simulink model "cddDcmApp"  (R2022b)
settings: Solver=FixedStepDiscrete  StartTime=0.0  StopTime=10.0  FixedStep=0.02  RelTol=1e-3
undefined symbols (not defined in the file or in its folder): CCPFaultType, DcChlType, HFAChlType, IcuChannelType, MtrRoutStaEnum, P_SignalPartType, P_Std_ReturnType, PowerChlType, SrsAdChlType, VGAChlType
(part 5 of 13)

== system cddDcmApp/cddDcmApp_Init_1 ==
  Event Listener : EventListener  EventName=reset

== system cddDcmApp/cddDcmApp_Init_sys ==
  function : TriggerPort  TriggerType=function-call  VariantControl=(inherit)

== system cddDcmApp/DataServices_DID_4756_DID_4756_Front_Mosfet_Blower_FB/DataServices_DID_4756_DID_4756_Front_Mosfet_Blower_FB_Voltage_sys ==
  function : TriggerPort  TriggerType=function-call  IsSimulinkFunction=on  FunctionName=DataServices_DID_4756_DID_4756_Front_Mosfet_Blower_FB_Voltage  VariantControl=(inherit)
  Ground_1 : Ground
  Ground_2 : Ground
  Terminator_1 : Terminator
  OpStatus : ArgIn  OutDataTypeStr=Enum: Dcm_OpStatusType  ArgumentName=OpStatus
  Data : ArgOut  OutDataTypeStr=uint8  ArgumentName=Data
  ERR : ArgOut  Port=2  OutDataTypeStr=Std_ReturnType  ArgumentName=ERR
  wires:
  OpStatus/1 -> Terminator_1/1
  Ground_1/1 -> Data/1
  Ground_2/1 -> ERR/1

== system cddDcmApp/DataServices_DID_47F3_DID_47F3_AIU_Failure_Reasons_and/DataServices_DID_47F3_DID_47F3_AIU_Failure_Reasons_and_Status_sys ==
  function : TriggerPort  TriggerType=function-call  IsSimulinkFunction=on  FunctionName=DataServices_DID_47F3_DID_47F3_AIU_Failure_Reasons_and_Status  VariantControl=(inherit)
  Ground_1 : Ground
  Ground_2 : Ground
  Terminator_1 : Terminator
  OpStatus : ArgIn  OutDataTypeStr=Enum: Dcm_OpStatusType  ArgumentName=OpStatus
  Data : ArgOut  OutDataTypeStr=uint8  ArgumentName=Data
  ERR : ArgOut  Port=2  OutDataTypeStr=Std_ReturnType  ArgumentName=ERR
  wires:
  OpStatus/1 -> Terminator_1/1
  Ground_1/1 -> Data/1
  Ground_2/1 -> ERR/1

== system cddDcmApp/DataServices_DID_47F4_DID_47F4_AQS_Air_Quality/DataServices_DID_47F4_DID_47F4_AQS_Air_Quality_Level_sys ==
  function : TriggerPort  TriggerType=function-call  IsSimulinkFunction=on  FunctionName=DataServices_DID_47F4_DID_47F4_AQS_Air_Quality_Level  VariantControl=(inherit)
  Ground_1 : Ground
  Ground_2 : Ground
  Terminator_1 : Terminator
  OpStatus : ArgIn  OutDataTypeStr=Enum: Dcm_OpStatusType  ArgumentName=OpStatus
  Data : ArgOut  OutDataTypeStr=uint8  ArgumentName=Data
  ERR : ArgOut  Port=2  OutDataTypeStr=Std_ReturnType  ArgumentName=ERR
  wires:
  OpStatus/1 -> Terminator_1/1
  Ground_1/1 -> Data/1
  Ground_2/1 -> ERR/1

== system cddDcmApp/DataServices_DID_47F5_DID_47F5_TestRunCriteria_TRC/DataServices_DID_47F5_DID_47F5_TestRunCriteria_TRC_Result_sys ==
  function : TriggerPort  TriggerType=function-call  IsSimulinkFunction=on  FunctionName=DataServices_DID_47F5_DID_47F5_TestRunCriteria_TRC_Result  VariantControl=(inherit)
  Ground_1 : Ground
  Ground_2 : Ground
  Terminator_1 : Terminator
  OpStatus : ArgIn  OutDataTypeStr=Enum: Dcm_OpStatusType  ArgumentName=OpStatus
  Data : ArgOut  OutDataTypeStr=uint8  ArgumentName=Data
  ERR : ArgOut  Port=2  OutDataTypeStr=Std_ReturnType  ArgumentName=ERR
  wires:
  OpStatus/1 -> Terminator_1/1
  Ground_1/1 -> Data/1
  Ground_2/1 -> ERR/1

== system cddDcmApp/DataServices_DID_47F6_DID_47F6_CCM_Lin1_Frame/DataServices_DID_47F6_DID_47F6_CCM_Lin1_Frame_Status_sys ==
  function : TriggerPort  TriggerType=function-call  IsSimulinkFunction=on  FunctionName=DataServices_DID_47F6_DID_47F6_CCM_Lin1_Frame_Status  VariantControl=(inherit)
  Ground_1 : Ground
  Ground_2 : Ground
  Terminator_1 : Terminator
  OpStatus : ArgIn  OutDataTypeStr=Enum: Dcm_OpStatusType  ArgumentName=OpStatus
  Data : ArgOut  OutDataTypeStr=uint8  ArgumentName=Data
  ERR : ArgOut  Port=2  OutDataTypeStr=Std_ReturnType  ArgumentName=ERR
  wires:
  OpStatus/1 -> Terminator_1/1
  Ground_1/1 -> Data/1
  Ground_2/1 -> ERR/1

== system cddDcmApp/DataServices_DID_47F7_DID_47F7_CCM_Lin2_Frame/DataServices_DID_47F7_DID_47F7_CCM_Lin2_Frame_Status_sys ==
  function : TriggerPort  TriggerType=function-call  IsSimulinkFunction=on  FunctionName=DataServices_DID_47F7_DID_47F7_CCM_Lin2_Frame_Status  VariantControl=(inherit)
  Ground_1 : Ground
  Ground_2 : Ground
  Terminator_1 : Terminator
  OpStatus : ArgIn  OutDataTypeStr=Enum: Dcm_OpStatusType  ArgumentName=OpStatus
  Data : ArgOut  OutDataTypeStr=uint8  ArgumentName=Data
  ERR : ArgOut  Port=2  OutDataTypeStr=Std_ReturnType  ArgumentName=ERR
  wires:
  OpStatus/1 -> Terminator_1/1
  Ground_1/1 -> Data/1
  Ground_2/1 -> ERR/1

== system cddDcmApp/DataServices_DID_47F8_DID_47F8_CCM_Lin3_Frame/DataServices_DID_47F8_DID_47F8_CCM_Lin3_Frame_Status_sys ==
  function : TriggerPort  TriggerType=function-call  IsSimulinkFunction=on  FunctionName=DataServices_DID_47F8_DID_47F8_CCM_Lin3_Frame_Status  VariantControl=(inherit)
  Ground_1 : Ground
  Ground_2 : Ground
  Terminator_1 : Terminator
  OpStatus : ArgIn  OutDataTypeStr=Enum: Dcm_OpStatusType  ArgumentName=OpStatus
  Data : ArgOut  OutDataTypeStr=uint8  ArgumentName=Data
  ERR : ArgOut  Port=2  OutDataTypeStr=Std_ReturnType  ArgumentName=ERR
  wires:
  OpStatus/1 -> Terminator_1/1
  Ground_1/1 -> Data/1
  Ground_2/1 -> ERR/1

== system cddDcmApp/DataServices_DID_47F9_DID_47F9_Blower_Max_Level_Voltage/DataServices_DID_47F9_DID_47F9_Blower_Max_Level_Voltage_Limit_sys ==
  function : TriggerPort  TriggerType=function-call  IsSimulinkFunction=on  FunctionName=DataServices_DID_47F9_DID_47F9_Blower_Max_Level_Voltage_Limit  VariantControl=(inherit)
  Ground_1 : Ground
  Ground_2 : Ground
  Terminator_1 : Terminator
  OpStatus : ArgIn  OutDataTypeStr=Enum: Dcm_OpStatusType  ArgumentName=OpStatus
  Data : ArgOut  OutDataTypeStr=uint8  ArgumentName=Data
  ERR : ArgOut  Port=2  OutDataTypeStr=Std_ReturnType  ArgumentName=ERR
  wires:
  OpStatus/1 -> Terminator_1/1
  Ground_1/1 -> Data/1
  Ground_2/1 -> ERR/1

== system cddDcmApp/DataServices_DID_4AF7_DID_4AF7_1_DC_Motor_Drive_Request_Number/DataServices_DID_4AF7_DID_4AF7_1_DC_Motor_Drive_Request_Number_FreezeCurrentState_sys ==
  function : TriggerPort  TriggerType=function-call  IsSimulinkFunction=on  FunctionName=DataServices_DID_4AF7_DID_4AF7_1_DC_Motor_Drive_Request_Number_FreezeCurrentState  VariantControl=(inherit)
  Ground_1 : Ground
  Ground_2 : Ground
  Terminator_1 : Terminator
  OpStatus : ArgIn  OutDataTypeStr=Enum: Dcm_OpStatusType  ArgumentName=OpStatus
  ErrorCode : ArgOut  OutDataTypeStr=Enum: Dcm_NegativeResponseCodeType  ArgumentName=ErrorCode
  ERR : ArgOut  Port=2  OutDataTypeStr=Std_ReturnType  ArgumentName=ERR
  wires:
  OpStatus/1 -> Terminator_1/1
  Ground_1/1 -> ErrorCode/1
  Ground_2/1 -> ERR/1

== system cddDcmApp/DataServices_DID_4AF7_DID_4AF7_1_DC_Motor_Drive_Request_Number/DataServices_DID_4AF7_DID_4AF7_1_DC_Motor_Drive_Request_Number_ReadData_sys ==
  function : TriggerPort  TriggerType=function-call  IsSimulinkFunction=on  FunctionName=DataServices_DID_4AF7_DID_4AF7_1_DC_Motor_Drive_Request_Number_ReadData  VariantControl=(inherit)
  Ground_1 : Ground
  Ground_2 : Ground
  Terminator_1 : Terminator
  OpStatus : ArgIn  OutDataTypeStr=Enum: Dcm_OpStatusType  ArgumentName=OpStatus
  Data : ArgOut  OutDataTypeStr=uint8  ArgumentName=Data
  ERR : ArgOut  Port=2  OutDataTypeStr=Std_ReturnType  ArgumentName=ERR
  wires:
  OpStatus/1 -> Terminator_1/1
  Ground_1/1 -> Data/1
  Ground_2/1 -> ERR/1

== system cddDcmApp/DataServices_DID_4AF7_DID_4AF7_1_DC_Motor_Drive_Request_Number/DataServices_DID_4AF7_DID_4AF7_1_DC_Motor_Drive_Request_Number_ReturnControlToECU_sys ==
  function : TriggerPort  TriggerType=function-call  IsSimulinkFunction=on  FunctionName=DataServices_DID_4AF7_DID_4AF7_1_DC_Motor_Drive_Request_Number_ReturnControlToECU  VariantControl=(inherit)
  Ground_1 : Ground
  Ground_2 : Ground
  ErrorCode : ArgOut  OutDataTypeStr=Enum: Dcm_NegativeResponseCodeType  ArgumentName=ErrorCode
  ERR : ArgOut  Port=2  OutDataTypeStr=Std_ReturnType  ArgumentName=ERR
  wires:
  Ground_1/1 -> ErrorCode/1
  Ground_2/1 -> ERR/1

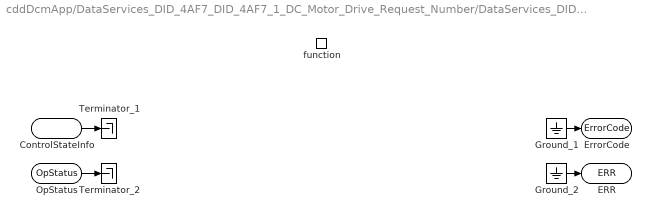
== system cddDcmApp/DataServices_DID_4AF7_DID_4AF7_1_DC_Motor_Drive_Request_Number/DataServices_DID_4AF7_DID_4AF7_1_DC_Motor_Drive_Request_Number_ShortTermAdjustment_sys ==
  function : TriggerPort  TriggerType=function-call  IsSimulinkFunction=on  FunctionName=DataServices_DID_4AF7_DID_4AF7_1_DC_Motor_Drive_Request_Number_ShortTermAdjustment  VariantControl=(inherit)
  Ground_1 : Ground
  Ground_2 : Ground
  Terminator_1 : Terminator
  Terminator_2 : Terminator
  ControlStateInfo : ArgIn  OutDataTypeStr=uint8  ArgumentName=ControlStateInfo
  OpStatus : ArgIn  Port=2  OutDataTypeStr=Enum: Dcm_OpStatusType  ArgumentName=OpStatus
  ErrorCode : ArgOut  OutDataTypeStr=Enum: Dcm_NegativeResponseCodeType  ArgumentName=ErrorCode
  ERR : ArgOut  Port=2  OutDataTypeStr=Std_ReturnType  ArgumentName=ERR
  wires:
  ControlStateInfo/1 -> Terminator_1/1
  OpStatus/1 -> Terminator_2/1
  Ground_1/1 -> ErrorCode/1
  Ground_2/1 -> ERR/1

== system cddDcmApp/DataServices_DID_4AF7_DID_4AF7_2_DC_Motor_Drive_Request_Time/DataServices_DID_4AF7_DID_4AF7_2_DC_Motor_Drive_Request_Time_FreezeCurrentState_sys ==
  function : TriggerPort  TriggerType=function-call  IsSimulinkFunction=on  FunctionName=DataServices_DID_4AF7_DID_4AF7_2_DC_Motor_Drive_Request_Time_FreezeCurrentState  VariantControl=(inherit)
  Ground_1 : Ground
  Ground_2 : Ground
  Terminator_1 : Terminator
  OpStatus : ArgIn  OutDataTypeStr=Enum: Dcm_OpStatusType  ArgumentName=OpStatus
  ErrorCode : ArgOut  OutDataTypeStr=Enum: Dcm_NegativeResponseCodeType  ArgumentName=ErrorCode
  ERR : ArgOut  Port=2  OutDataTypeStr=Std_ReturnType  ArgumentName=ERR
  wires:
  OpStatus/1 -> Terminator_1/1
  Ground_1/1 -> ErrorCode/1
  Ground_2/1 -> ERR/1

== system cddDcmApp/DataServices_DID_4AF7_DID_4AF7_2_DC_Motor_Drive_Request_Time/DataServices_DID_4AF7_DID_4AF7_2_DC_Motor_Drive_Request_Time_ReadData_sys ==
  function : TriggerPort  TriggerType=function-call  IsSimulinkFunction=on  FunctionName=DataServices_DID_4AF7_DID_4AF7_2_DC_Motor_Drive_Request_Time_ReadData  VariantControl=(inherit)
  Ground_1 : Ground
  Ground_2 : Ground
  Terminator_1 : Terminator
  OpStatus : ArgIn  OutDataTypeStr=Enum: Dcm_OpStatusType  ArgumentName=OpStatus
  Data : ArgOut  OutDataTypeStr=uint8  ArgumentName=Data
  ERR : ArgOut  Port=2  OutDataTypeStr=Std_ReturnType  ArgumentName=ERR
  wires:
  OpStatus/1 -> Terminator_1/1
  Ground_1/1 -> Data/1
  Ground_2/1 -> ERR/1

== system cddDcmApp/DataServices_DID_4AF7_DID_4AF7_2_DC_Motor_Drive_Request_Time/DataServices_DID_4AF7_DID_4AF7_2_DC_Motor_Drive_Request_Time_ReturnControlToECU_sys ==
  function : TriggerPort  TriggerType=function-call  IsSimulinkFunction=on  FunctionName=DataServices_DID_4AF7_DID_4AF7_2_DC_Motor_Drive_Request_Time_ReturnControlToECU  VariantControl=(inherit)
  Ground_1 : Ground
  Ground_2 : Ground
  ErrorCode : ArgOut  OutDataTypeStr=Enum: Dcm_NegativeResponseCodeType  ArgumentName=ErrorCode
  ERR : ArgOut  Port=2  OutDataTypeStr=Std_ReturnType  ArgumentName=ERR
  wires:
  Ground_1/1 -> ErrorCode/1
  Ground_2/1 -> ERR/1

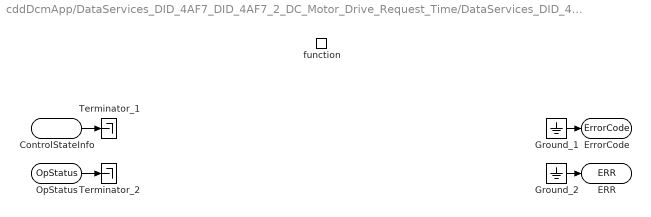
== system cddDcmApp/DataServices_DID_4AF7_DID_4AF7_2_DC_Motor_Drive_Request_Time/DataServices_DID_4AF7_DID_4AF7_2_DC_Motor_Drive_Request_Time_ShortTermAdjustment_sys ==
  function : TriggerPort  TriggerType=function-call  IsSimulinkFunction=on  FunctionName=DataServices_DID_4AF7_DID_4AF7_2_DC_Motor_Drive_Request_Time_ShortTermAdjustment  VariantControl=(inherit)
  Ground_1 : Ground
  Ground_2 : Ground
  Terminator_1 : Terminator
  Terminator_2 : Terminator
  ControlStateInfo : ArgIn  OutDataTypeStr=uint8  ArgumentName=ControlStateInfo
  OpStatus : ArgIn  Port=2  OutDataTypeStr=Enum: Dcm_OpStatusType  ArgumentName=OpStatus
  ErrorCode : ArgOut  OutDataTypeStr=Enum: Dcm_NegativeResponseCodeType  ArgumentName=ErrorCode
  ERR : ArgOut  Port=2  OutDataTypeStr=Std_ReturnType  ArgumentName=ERR
  wires:
  ControlStateInfo/1 -> Terminator_1/1
  OpStatus/1 -> Terminator_2/1
  Ground_1/1 -> ErrorCode/1
  Ground_2/1 -> ERR/1

== system cddDcmApp/DataServices_DID_4AF7_DID_4AF7_3_DC_Motor_Drive_Request_Direction/DataServices_DID_4AF7_DID_4AF7_3_DC_Motor_Drive_Request_Direction_FreezeCurrentState_sys ==
  function : TriggerPort  TriggerType=function-call  IsSimulinkFunction=on  FunctionName=DataServices_DID_4AF7_DID_4AF7_3_DC_Motor_Drive_Request_Direction_FreezeCurrentState  VariantControl=(inherit)
  Ground_1 : Ground
  Ground_2 : Ground
  Terminator_1 : Terminator
  OpStatus : ArgIn  OutDataTypeStr=Enum: Dcm_OpStatusType  ArgumentName=OpStatus
  ErrorCode : ArgOut  OutDataTypeStr=Enum: Dcm_NegativeResponseCodeType  ArgumentName=ErrorCode
  ERR : ArgOut  Port=2  OutDataTypeStr=Std_ReturnType  ArgumentName=ERR
  wires:
  OpStatus/1 -> Terminator_1/1
  Ground_1/1 -> ErrorCode/1
  Ground_2/1 -> ERR/1

== system cddDcmApp/DataServices_DID_4AF7_DID_4AF7_3_DC_Motor_Drive_Request_Direction/DataServices_DID_4AF7_DID_4AF7_3_DC_Motor_Drive_Request_Direction_ReadData_sys ==
  function : TriggerPort  TriggerType=function-call  IsSimulinkFunction=on  FunctionName=DataServices_DID_4AF7_DID_4AF7_3_DC_Motor_Drive_Request_Direction_ReadData  VariantControl=(inherit)
  Ground_1 : Ground
  Ground_2 : Ground
  Terminator_1 : Terminator
  OpStatus : ArgIn  OutDataTypeStr=Enum: Dcm_OpStatusType  ArgumentName=OpStatus
  Data : ArgOut  OutDataTypeStr=uint8  ArgumentName=Data
  ERR : ArgOut  Port=2  OutDataTypeStr=Std_ReturnType  ArgumentName=ERR
  wires:
  OpStatus/1 -> Terminator_1/1
  Ground_1/1 -> Data/1
  Ground_2/1 -> ERR/1

== system cddDcmApp/DataServices_DID_4AF7_DID_4AF7_3_DC_Motor_Drive_Request_Direction/DataServices_DID_4AF7_DID_4AF7_3_DC_Motor_Drive_Request_Direction_ReturnControlToECU_sys ==
  function : TriggerPort  TriggerType=function-call  IsSimulinkFunction=on  FunctionName=DataServices_DID_4AF7_DID_4AF7_3_DC_Motor_Drive_Request_Direction_ReturnControlToECU  VariantControl=(inherit)
  Ground_1 : Ground
  Ground_2 : Ground
  ErrorCode : ArgOut  OutDataTypeStr=Enum: Dcm_NegativeResponseCodeType  ArgumentName=ErrorCode
  ERR : ArgOut  Port=2  OutDataTypeStr=Std_ReturnType  ArgumentName=ERR
  wires:
  Ground_1/1 -> ErrorCode/1
  Ground_2/1 -> ERR/1

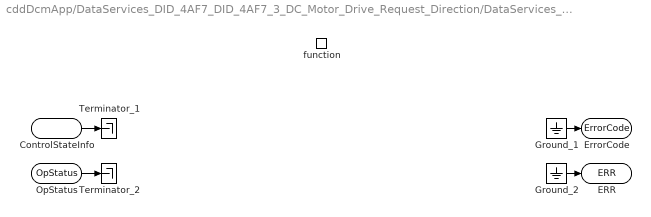
== system cddDcmApp/DataServices_DID_4AF7_DID_4AF7_3_DC_Motor_Drive_Request_Direction/DataServices_DID_4AF7_DID_4AF7_3_DC_Motor_Drive_Request_Direction_ShortTermAdjustment_sys ==
  function : TriggerPort  TriggerType=function-call  IsSimulinkFunction=on  FunctionName=DataServices_DID_4AF7_DID_4AF7_3_DC_Motor_Drive_Request_Direction_ShortTermAdjustment  VariantControl=(inherit)
  Ground_1 : Ground
  Ground_2 : Ground
  Terminator_1 : Terminator
  Terminator_2 : Terminator
  ControlStateInfo : ArgIn  OutDataTypeStr=uint8  ArgumentName=ControlStateInfo
  OpStatus : ArgIn  Port=2  OutDataTypeStr=Enum: Dcm_OpStatusType  ArgumentName=OpStatus
  ErrorCode : ArgOut  OutDataTypeStr=Enum: Dcm_NegativeResponseCodeType  ArgumentName=ErrorCode
  ERR : ArgOut  Port=2  OutDataTypeStr=Std_ReturnType  ArgumentName=ERR
  wires:
  ControlStateInfo/1 -> Terminator_1/1
  OpStatus/1 -> Terminator_2/1
  Ground_1/1 -> ErrorCode/1
  Ground_2/1 -> ERR/1

== system cddDcmApp/DataServices_DID_9800_DID_9800_1_Evaporator_Temperature/DataServices_DID_9800_DID_9800_1_Evaporator_Temperature_ReadData_sys ==
  function : TriggerPort  TriggerType=function-call  IsSimulinkFunction=on  FunctionName=DataServices_DID_9800_DID_9800_1_Evaporator_Temperature_ReadData  VariantControl=(inherit)
  Ground_1 : Ground
  Ground_2 : Ground
  Terminator_1 : Terminator
  OpStatus : ArgIn  OutDataTypeStr=Enum: Dcm_OpStatusType  ArgumentName=OpStatus
  Data : ArgOut  OutDataTypeStr=uint8  ArgumentName=Data
  ERR : ArgOut  Port=2  OutDataTypeStr=Std_ReturnType  ArgumentName=ERR
  wires:
  OpStatus/1 -> Terminator_1/1
  Ground_1/1 -> Data/1
  Ground_2/1 -> ERR/1

== system cddDcmApp/DataServices_DID_9800_DID_9800_2_incar_temperature/DataServices_DID_9800_DID_9800_2_incar_temperature_ReadData_sys ==
  function : TriggerPort  TriggerType=function-call  IsSimulinkFunction=on  FunctionName=DataServices_DID_9800_DID_9800_2_incar_temperature_ReadData  VariantControl=(inherit)
  Ground_1 : Ground
  Ground_2 : Ground
  Terminator_1 : Terminator
  OpStatus : ArgIn  OutDataTypeStr=Enum: Dcm_OpStatusType  ArgumentName=OpStatus
  Data : ArgOut  OutDataTypeStr=uint8  ArgumentName=Data
  ERR : ArgOut  Port=2  OutDataTypeStr=Std_ReturnType  ArgumentName=ERR
  wires:
  OpStatus/1 -> Terminator_1/1
  Ground_1/1 -> Data/1
  Ground_2/1 -> ERR/1

== system cddDcmApp/DataServices_DID_9800_DID_9800_3_outside_temperature/DataServices_DID_9800_DID_9800_3_outside_temperature_ReadData_sys ==
  function : TriggerPort  TriggerType=function-call  IsSimulinkFunction=on  FunctionName=DataServices_DID_9800_DID_9800_3_outside_temperature_ReadData  VariantControl=(inherit)
  Ground_1 : Ground
  Ground_2 : Ground
  Terminator_1 : Terminator
  OpStatus : ArgIn  OutDataTypeStr=Enum: Dcm_OpStatusType  ArgumentName=OpStatus
  Data : ArgOut  OutDataTypeStr=uint8  ArgumentName=Data
  ERR : ArgOut  Port=2  OutDataTypeStr=Std_ReturnType  ArgumentName=ERR
  wires:
  OpStatus/1 -> Terminator_1/1
  Ground_1/1 -> Data/1
  Ground_2/1 -> ERR/1

== system cddDcmApp/DataServices_DID_9800_DID_9800_4_Hex_sensor/DataServices_DID_9800_DID_9800_4_Hex_sensor_ReadData_sys ==
  function : TriggerPort  TriggerType=function-call  IsSimulinkFunction=on  FunctionName=DataServices_DID_9800_DID_9800_4_Hex_sensor_ReadData  VariantControl=(inherit)
  Ground_1 : Ground
  Ground_2 : Ground
  Terminator_1 : Terminator
  OpStatus : ArgIn  OutDataTypeStr=Enum: Dcm_OpStatusType  ArgumentName=OpStatus
  Data : ArgOut  OutDataTypeStr=uint8  ArgumentName=Data
  ERR : ArgOut  Port=2  OutDataTypeStr=Std_ReturnType  ArgumentName=ERR
  wires:
  OpStatus/1 -> Terminator_1/1
  Ground_1/1 -> Data/1
  Ground_2/1 -> ERR/1

== system cddDcmApp/DataServices_DID_9801_DID_9801_1_Front_left_vent_temperature/DataServices_DID_9801_DID_9801_1_Front_left_vent_temperature_ReadData_sys ==
  function : TriggerPort  TriggerType=function-call  IsSimulinkFunction=on  FunctionName=DataServices_DID_9801_DID_9801_1_Front_left_vent_temperature_ReadData  VariantControl=(inherit)
  Ground_1 : Ground
  Ground_2 : Ground
  Terminator_1 : Terminator
  OpStatus : ArgIn  OutDataTypeStr=Enum: Dcm_OpStatusType  ArgumentName=OpStatus
  Data : ArgOut  OutDataTypeStr=uint8  ArgumentName=Data
  ERR : ArgOut  Port=2  OutDataTypeStr=Std_ReturnType  ArgumentName=ERR
  wires:
  OpStatus/1 -> Terminator_1/1
  Ground_1/1 -> Data/1
  Ground_2/1 -> ERR/1

== system cddDcmApp/DataServices_DID_9801_DID_9801_2_Front_left_floor_temperature/DataServices_DID_9801_DID_9801_2_Front_left_floor_temperature_ReadData_sys ==
  function : TriggerPort  TriggerType=function-call  IsSimulinkFunction=on  FunctionName=DataServices_DID_9801_DID_9801_2_Front_left_floor_temperature_ReadData  VariantControl=(inherit)
  Ground_1 : Ground
  Ground_2 : Ground
  Terminator_1 : Terminator
  OpStatus : ArgIn  OutDataTypeStr=Enum: Dcm_OpStatusType  ArgumentName=OpStatus
  Data : ArgOut  OutDataTypeStr=uint8  ArgumentName=Data
  ERR : ArgOut  Port=2  OutDataTypeStr=Std_ReturnType  ArgumentName=ERR
  wires:
  OpStatus/1 -> Terminator_1/1
  Ground_1/1 -> Data/1
  Ground_2/1 -> ERR/1

== system cddDcmApp/DataServices_DID_9801_DID_9801_3_Front_right_vent_temperature/DataServices_DID_9801_DID_9801_3_Front_right_vent_temperature_ReadData_sys ==
  function : TriggerPort  TriggerType=function-call  IsSimulinkFunction=on  FunctionName=DataServices_DID_9801_DID_9801_3_Front_right_vent_temperature_ReadData  VariantControl=(inherit)
  Ground_1 : Ground
  Ground_2 : Ground
  Terminator_1 : Terminator
  OpStatus : ArgIn  OutDataTypeStr=Enum: Dcm_OpStatusType  ArgumentName=OpStatus
  Data : ArgOut  OutDataTypeStr=uint8  ArgumentName=Data
  ERR : ArgOut  Port=2  OutDataTypeStr=Std_ReturnType  ArgumentName=ERR
  wires:
  OpStatus/1 -> Terminator_1/1
  Ground_1/1 -> Data/1
  Ground_2/1 -> ERR/1

== system cddDcmApp/DataServices_DID_9801_DID_9801_4_Front_right_floor_temperature/DataServices_DID_9801_DID_9801_4_Front_right_floor_temperature_ReadData_sys ==
  function : TriggerPort  TriggerType=function-call  IsSimulinkFunction=on  FunctionName=DataServices_DID_9801_DID_9801_4_Front_right_floor_temperature_ReadData  VariantControl=(inherit)
  Ground_1 : Ground
  Ground_2 : Ground
  Terminator_1 : Terminator
  OpStatus : ArgIn  OutDataTypeStr=Enum: Dcm_OpStatusType  ArgumentName=OpStatus
  Data : ArgOut  OutDataTypeStr=uint8  ArgumentName=Data
  ERR : ArgOut  Port=2  OutDataTypeStr=Std_ReturnType  ArgumentName=ERR
  wires:
  OpStatus/1 -> Terminator_1/1
  Ground_1/1 -> Data/1
  Ground_2/1 -> ERR/1

== system cddDcmApp/DataServices_DID_9802_1_Second_row_left_vent_temperatureSecond_row_vent_temperature/DataServices_DID_9802_1_Second_row_left_vent_temperatureSecond_row_vent_temperature_ReadData_sys ==
  function : TriggerPort  TriggerType=function-call  IsSimulinkFunction=on  FunctionName=DataServices_DID_9802_1_Second_row_left_vent_temperatureSecond_row_vent_temperature_ReadData  VariantControl=(inherit)
  Ground_1 : Ground
  Ground_2 : Ground
  Terminator_1 : Terminator
  OpStatus : ArgIn  OutDataTypeStr=Enum: Dcm_OpStatusType  ArgumentName=OpStatus
  Data : ArgOut  OutDataTypeStr=uint8  ArgumentName=Data
  ERR : ArgOut  Port=2  OutDataTypeStr=Std_ReturnType  ArgumentName=ERR
  wires:
  OpStatus/1 -> Terminator_1/1
  Ground_1/1 -> Data/1
  Ground_2/1 -> ERR/1

== system cddDcmApp/DataServices_DID_9802_2_Second_row_left_floor_temperatureSecond_row_floor_temperature/DataServices_DID_9802_2_Second_row_left_floor_temperatureSecond_row_floor_temperature_ReadData_sys ==
  function : TriggerPort  TriggerType=function-call  IsSimulinkFunction=on  FunctionName=DataServices_DID_9802_2_Second_row_left_floor_temperatureSecond_row_floor_temperature_ReadData  VariantControl=(inherit)
  Ground_1 : Ground
  Ground_2 : Ground
  Terminator_1 : Terminator
  OpStatus : ArgIn  OutDataTypeStr=Enum: Dcm_OpStatusType  ArgumentName=OpStatus
  Data : ArgOut  OutDataTypeStr=uint8  ArgumentName=Data
  ERR : ArgOut  Port=2  OutDataTypeStr=Std_ReturnType  ArgumentName=ERR
  wires:
  OpStatus/1 -> Terminator_1/1
  Ground_1/1 -> Data/1
  Ground_2/1 -> ERR/1

== system cddDcmApp/DataServices_DID_9802_DID_9802_3_Second_row_right_vent_temperature/DataServices_DID_9802_DID_9802_3_Second_row_right_vent_temperature_ReadData_sys ==
  function : TriggerPort  TriggerType=function-call  IsSimulinkFunction=on  FunctionName=DataServices_DID_9802_DID_9802_3_Second_row_right_vent_temperature_ReadData  VariantControl=(inherit)
  Ground_1 : Ground
  Ground_2 : Ground
  Terminator_1 : Terminator
  OpStatus : ArgIn  OutDataTypeStr=Enum: Dcm_OpStatusType  ArgumentName=OpStatus
  Data : ArgOut  OutDataTypeStr=uint8  ArgumentName=Data
  ERR : ArgOut  Port=2  OutDataTypeStr=Std_ReturnType  ArgumentName=ERR
  wires:
  OpStatus/1 -> Terminator_1/1
  Ground_1/1 -> Data/1
  Ground_2/1 -> ERR/1

== system cddDcmApp/DataServices_DID_9802_DID_9802_4_Second_row_right_floor_temperature/DataServices_DID_9802_DID_9802_4_Second_row_right_floor_temperature_ReadData_sys ==
  function : TriggerPort  TriggerType=function-call  IsSimulinkFunction=on  FunctionName=DataServices_DID_9802_DID_9802_4_Second_row_right_floor_temperature_ReadData  VariantControl=(inherit)
  Ground_1 : Ground
  Ground_2 : Ground
  Terminator_1 : Terminator
  OpStatus : ArgIn  OutDataTypeStr=Enum: Dcm_OpStatusType  ArgumentName=OpStatus
  Data : ArgOut  OutDataTypeStr=uint8  ArgumentName=Data
  ERR : ArgOut  Port=2  OutDataTypeStr=Std_ReturnType  ArgumentName=ERR
  wires:
  OpStatus/1 -> Terminator_1/1
  Ground_1/1 -> Data/1
  Ground_2/1 -> ERR/1

== system cddDcmApp/DataServices_DID_9803_DID_9803_1_Left_sun_intensity/DataServices_DID_9803_DID_9803_1_Left_sun_intensity_ReadData_sys ==
  function : TriggerPort  TriggerType=function-call  IsSimulinkFunction=on  FunctionName=DataServices_DID_9803_DID_9803_1_Left_sun_intensity_ReadData  VariantControl=(inherit)
  Ground_1 : Ground
  Ground_2 : Ground
  Terminator_1 : Terminator
  OpStatus : ArgIn  OutDataTypeStr=Enum: Dcm_OpStatusType  ArgumentName=OpStatus
  Data : ArgOut  OutDataTypeStr=uint8  ArgumentName=Data
  ERR : ArgOut  Port=2  OutDataTypeStr=Std_ReturnType  ArgumentName=ERR
  wires:
  OpStatus/1 -> Terminator_1/1
  Ground_1/1 -> Data/1
  Ground_2/1 -> ERR/1

== system cddDcmApp/DataServices_DID_9803_DID_9803_2_Right_sun_intensity/DataServices_DID_9803_DID_9803_2_Right_sun_intensity_ReadData_sys ==
  function : TriggerPort  TriggerType=function-call  IsSimulinkFunction=on  FunctionName=DataServices_DID_9803_DID_9803_2_Right_sun_intensity_ReadData  VariantControl=(inherit)
  Ground_1 : Ground
  Ground_2 : Ground
  Terminator_1 : Terminator
  OpStatus : ArgIn  OutDataTypeStr=Enum: Dcm_OpStatusType  ArgumentName=OpStatus
  Data : ArgOut  OutDataTypeStr=uint8  ArgumentName=Data
  ERR : ArgOut  Port=2  OutDataTypeStr=Std_ReturnType  ArgumentName=ERR
  wires:
  OpStatus/1 -> Terminator_1/1
  Ground_1/1 -> Data/1
  Ground_2/1 -> ERR/1

== system cddDcmApp/DataServices_DID_9803_DID_9803_3_engine_coolant_temperature/DataServices_DID_9803_DID_9803_3_engine_coolant_temperature_ReadData_sys ==
  function : TriggerPort  TriggerType=function-call  IsSimulinkFunction=on  FunctionName=DataServices_DID_9803_DID_9803_3_engine_coolant_temperature_ReadData  VariantControl=(inherit)
  Ground_1 : Ground
  Ground_2 : Ground
  Terminator_1 : Terminator
  OpStatus : ArgIn  OutDataTypeStr=Enum: Dcm_OpStatusType  ArgumentName=OpStatus
  Data : ArgOut  OutDataTypeStr=uint8  ArgumentName=Data
  ERR : ArgOut  Port=2  OutDataTypeStr=Std_ReturnType  ArgumentName=ERR
  wires:
  OpStatus/1 -> Terminator_1/1
  Ground_1/1 -> Data/1
  Ground_2/1 -> ERR/1

== system cddDcmApp/DataServices_DID_9803_DID_9803_4_dew_temperature/DataServices_DID_9803_DID_9803_4_dew_temperature_ReadData_sys ==
  function : TriggerPort  TriggerType=function-call  IsSimulinkFunction=on  FunctionName=DataServices_DID_9803_DID_9803_4_dew_temperature_ReadData  VariantControl=(inherit)
  Ground_1 : Ground
  Ground_2 : Ground
  Terminator_1 : Terminator
  OpStatus : ArgIn  OutDataTypeStr=Enum: Dcm_OpStatusType  ArgumentName=OpStatus
  Data : ArgOut  OutDataTypeStr=uint8  ArgumentName=Data
  ERR : ArgOut  Port=2  OutDataTypeStr=Std_ReturnType  ArgumentName=ERR
  wires:
  OpStatus/1 -> Terminator_1/1
  Ground_1/1 -> Data/1
  Ground_2/1 -> ERR/1

== system cddDcmApp/DataServices_DID_9804_DID_9804_1_Relative_Humidity/DataServices_DID_9804_DID_9804_1_Relative_Humidity_ReadData_sys ==
  function : TriggerPort  TriggerType=function-call  IsSimulinkFunction=on  FunctionName=DataServices_DID_9804_DID_9804_1_Relative_Humidity_ReadData  VariantControl=(inherit)
  Ground_1 : Ground
  Ground_2 : Ground
  Terminator_1 : Terminator
  OpStatus : ArgIn  OutDataTypeStr=Enum: Dcm_OpStatusType  ArgumentName=OpStatus
  Data : ArgOut  OutDataTypeStr=uint8  ArgumentName=Data
  ERR : ArgOut  Port=2  OutDataTypeStr=Std_ReturnType  ArgumentName=ERR
  wires:
  OpStatus/1 -> Terminator_1/1
  Ground_1/1 -> Data/1
  Ground_2/1 -> ERR/1

== system cddDcmApp/DataServices_DID_9804_DID_9804_2_AQS_sensor/DataServices_DID_9804_DID_9804_2_AQS_sensor_ReadData_sys ==
  function : TriggerPort  TriggerType=function-call  IsSimulinkFunction=on  FunctionName=DataServices_DID_9804_DID_9804_2_AQS_sensor_ReadData  VariantControl=(inherit)
  Ground_1 : Ground
  Ground_2 : Ground
  Terminator_1 : Terminator
  OpStatus : ArgIn  OutDataTypeStr=Enum: Dcm_OpStatusType  ArgumentName=OpStatus
  Data : ArgOut  OutDataTypeStr=uint8  ArgumentName=Data
  ERR : ArgOut  Port=2  OutDataTypeStr=Std_ReturnType  ArgumentName=ERR
  wires:
  OpStatus/1 -> Terminator_1/1
  Ground_1/1 -> Data/1
  Ground_2/1 -> ERR/1

== system cddDcmApp/DataServices_DID_9804_DID_9804_3_PM25PM25_in_sensor_concentration/DataServices_DID_9804_DID_9804_3_PM25PM25_in_sensor_concentration_ReadData_sys ==
  function : TriggerPort  TriggerType=function-call  IsSimulinkFunction=on  FunctionName=DataServices_DID_9804_DID_9804_3_PM25PM25_in_sensor_concentration_ReadData  VariantControl=(inherit)
  Ground_1 : Ground
  Ground_2 : Ground
  Terminator_1 : Terminator
  OpStatus : ArgIn  OutDataTypeStr=Enum: Dcm_OpStatusType  ArgumentName=OpStatus
  Data : ArgOut  OutDataTypeStr=uint8  ArgumentName=Data
  ERR : ArgOut  Port=2  OutDataTypeStr=Std_ReturnType  ArgumentName=ERR
  wires:
  OpStatus/1 -> Terminator_1/1
  Ground_1/1 -> Data/1
  Ground_2/1 -> ERR/1

== system cddDcmApp/DataServices_DID_9804_DID_9804_4_PM25_out_sensor_concentration/DataServices_DID_9804_DID_9804_4_PM25_out_sensor_concentration_ReadData_sys ==
  function : TriggerPort  TriggerType=function-call  IsSimulinkFunction=on  FunctionName=DataServices_DID_9804_DID_9804_4_PM25_out_sensor_concentration_ReadData  VariantControl=(inherit)
  Ground_1 : Ground
  Ground_2 : Ground
  Terminator_1 : Terminator
  OpStatus : ArgIn  OutDataTypeStr=Enum: Dcm_OpStatusType  ArgumentName=OpStatus
  Data : ArgOut  OutDataTypeStr=uint8  ArgumentName=Data
  ERR : ArgOut  Port=2  OutDataTypeStr=Std_ReturnType  ArgumentName=ERR
  wires:
  OpStatus/1 -> Terminator_1/1
  Ground_1/1 -> Data/1
  Ground_2/1 -> ERR/1

== system cddDcmApp/DataServices_DID_9805_DID_9805_1_CO2_sensor_concentration/DataServices_DID_9805_DID_9805_1_CO2_sensor_concentration_ReadData_sys ==
  function : TriggerPort  TriggerType=function-call  IsSimulinkFunction=on  FunctionName=DataServices_DID_9805_DID_9805_1_CO2_sensor_concentration_ReadData  VariantControl=(inherit)
  Ground_1 : Ground
  Ground_2 : Ground
  Terminator_1 : Terminator
  OpStatus : ArgIn  OutDataTypeStr=Enum: Dcm_OpStatusType  ArgumentName=OpStatus
  Data : ArgOut  OutDataTypeStr=uint8  ArgumentName=Data
  ERR : ArgOut  Port=2  OutDataTypeStr=Std_ReturnType  ArgumentName=ERR
  wires:
  OpStatus/1 -> Terminator_1/1
  Ground_1/1 -> Data/1
  Ground_2/1 -> ERR/1

== system cddDcmApp/DataServices_DID_9805_DID_9805_2_Front_windscreen_temperature/DataServices_DID_9805_DID_9805_2_Front_windscreen_temperature_ReadData_sys ==
  function : TriggerPort  TriggerType=function-call  IsSimulinkFunction=on  FunctionName=DataServices_DID_9805_DID_9805_2_Front_windscreen_temperature_ReadData  VariantControl=(inherit)
  Ground_1 : Ground
  Ground_2 : Ground
  Terminator_1 : Terminator
  OpStatus : ArgIn  OutDataTypeStr=Enum: Dcm_OpStatusType  ArgumentName=OpStatus
  Data : ArgOut  OutDataTypeStr=uint8  ArgumentName=Data
  ERR : ArgOut  Port=2  OutDataTypeStr=Std_ReturnType  ArgumentName=ERR
  wires:
  OpStatus/1 -> Terminator_1/1
  Ground_1/1 -> Data/1
  Ground_2/1 -> ERR/1

== system cddDcmApp/DataServices_DID_9805_DID_9805_3_Rear_cabin_temperature/DataServices_DID_9805_DID_9805_3_Rear_cabin_temperature_ReadData_sys ==
  function : TriggerPort  TriggerType=function-call  IsSimulinkFunction=on  FunctionName=DataServices_DID_9805_DID_9805_3_Rear_cabin_temperature_ReadData  VariantControl=(inherit)
  Ground_1 : Ground
  Ground_2 : Ground
  Terminator_1 : Terminator
  OpStatus : ArgIn  OutDataTypeStr=Enum: Dcm_OpStatusType  ArgumentName=OpStatus
  Data : ArgOut  OutDataTypeStr=uint8  ArgumentName=Data
  ERR : ArgOut  Port=2  OutDataTypeStr=Std_ReturnType  ArgumentName=ERR
  wires:
  OpStatus/1 -> Terminator_1/1
  Ground_1/1 -> Data/1
  Ground_2/1 -> ERR/1

== system cddDcmApp/DataServices_DID_9805_DID_9805_4_Third_row_air_temperature/DataServices_DID_9805_DID_9805_4_Third_row_air_temperature_ReadData_sys ==
  function : TriggerPort  TriggerType=function-call  IsSimulinkFunction=on  FunctionName=DataServices_DID_9805_DID_9805_4_Third_row_air_temperature_ReadData  VariantControl=(inherit)
  Ground_1 : Ground
  Ground_2 : Ground
  Terminator_1 : Terminator
  OpStatus : ArgIn  OutDataTypeStr=Enum: Dcm_OpStatusType  ArgumentName=OpStatus
  Data : ArgOut  OutDataTypeStr=uint8  ArgumentName=Data
  ERR : ArgOut  Port=2  OutDataTypeStr=Std_ReturnType  ArgumentName=ERR
  wires:
  OpStatus/1 -> Terminator_1/1
  Ground_1/1 -> Data/1
  Ground_2/1 -> ERR/1

== system cddDcmApp/DataServices_DID_9808_DID_9808_RHFA_Actuator_Position/DataServices_DID_9808_DID_9808_RHFA_Actuator_Position_FreezeCurrentState_sys ==
  function : TriggerPort  TriggerType=function-call  IsSimulinkFunction=on  FunctionName=DataServices_DID_9808_DID_9808_RHFA_Actuator_Position_FreezeCurrentState  VariantControl=(inherit)
  Ground_1 : Ground
  Ground_2 : Ground
  Terminator_1 : Terminator
  OpStatus : ArgIn  OutDataTypeStr=Enum: Dcm_OpStatusType  ArgumentName=OpStatus
  ErrorCode : ArgOut  OutDataTypeStr=Enum: Dcm_NegativeResponseCodeType  ArgumentName=ErrorCode
  ERR : ArgOut  Port=2  OutDataTypeStr=Std_ReturnType  ArgumentName=ERR
  wires:
  OpStatus/1 -> Terminator_1/1
  Ground_1/1 -> ErrorCode/1
  Ground_2/1 -> ERR/1

== system cddDcmApp/DataServices_DID_9808_DID_9808_RHFA_Actuator_Position/DataServices_DID_9808_DID_9808_RHFA_Actuator_Position_ReadData_sys ==
  function : TriggerPort  TriggerType=function-call  IsSimulinkFunction=on  FunctionName=DataServices_DID_9808_DID_9808_RHFA_Actuator_Position_ReadData  VariantControl=(inherit)
  Ground_1 : Ground
  Ground_2 : Ground
  Terminator_1 : Terminator
  OpStatus : ArgIn  OutDataTypeStr=Enum: Dcm_OpStatusType  ArgumentName=OpStatus
  Data : ArgOut  OutDataTypeStr=uint8  ArgumentName=Data
  ERR : ArgOut  Port=2  OutDataTypeStr=Std_ReturnType  ArgumentName=ERR
  wires:
  OpStatus/1 -> Terminator_1/1
  Ground_1/1 -> Data/1
  Ground_2/1 -> ERR/1

== system cddDcmApp/DataServices_DID_9808_DID_9808_RHFA_Actuator_Position/DataServices_DID_9808_DID_9808_RHFA_Actuator_Position_ReturnControlToECU_sys ==
  function : TriggerPort  TriggerType=function-call  IsSimulinkFunction=on  FunctionName=DataServices_DID_9808_DID_9808_RHFA_Actuator_Position_ReturnControlToECU  VariantControl=(inherit)
  Ground_1 : Ground
  Ground_2 : Ground
  ErrorCode : ArgOut  OutDataTypeStr=Enum: Dcm_NegativeResponseCodeType  ArgumentName=ErrorCode
  ERR : ArgOut  Port=2  OutDataTypeStr=Std_ReturnType  ArgumentName=ERR
  wires:
  Ground_1/1 -> ErrorCode/1
  Ground_2/1 -> ERR/1

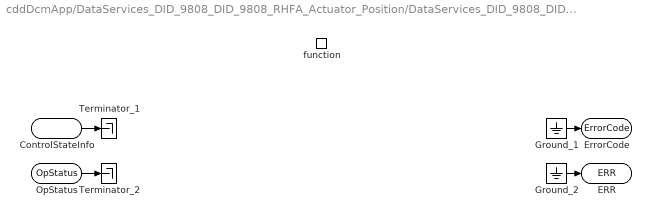
== system cddDcmApp/DataServices_DID_9808_DID_9808_RHFA_Actuator_Position/DataServices_DID_9808_DID_9808_RHFA_Actuator_Position_ShortTermAdjustment_sys ==
  function : TriggerPort  TriggerType=function-call  IsSimulinkFunction=on  FunctionName=DataServices_DID_9808_DID_9808_RHFA_Actuator_Position_ShortTermAdjustment  VariantControl=(inherit)
  Ground_1 : Ground
  Ground_2 : Ground
  Terminator_1 : Terminator
  Terminator_2 : Terminator
  ControlStateInfo : ArgIn  OutDataTypeStr=uint8  ArgumentName=ControlStateInfo
  OpStatus : ArgIn  Port=2  OutDataTypeStr=Enum: Dcm_OpStatusType  ArgumentName=OpStatus
  ErrorCode : ArgOut  OutDataTypeStr=Enum: Dcm_NegativeResponseCodeType  ArgumentName=ErrorCode
  ERR : ArgOut  Port=2  OutDataTypeStr=Std_ReturnType  ArgumentName=ERR
  wires:
  ControlStateInfo/1 -> Terminator_1/1
  OpStatus/1 -> Terminator_2/1
  Ground_1/1 -> ErrorCode/1
  Ground_2/1 -> ERR/1

== system cddDcmApp/DataServices_DID_9809_3_actuator_positionSecond_temperature_actuator_position/DataServices_DID_9809_3_actuator_positionSecond_temperature_actuator_position_FreezeCurrentState_sys ==
  function : TriggerPort  TriggerType=function-call  IsSimulinkFunction=on  FunctionName=DataServices_DID_9809_3_actuator_positionSecond_temperature_actuator_position_FreezeCurrentState  VariantControl=(inherit)
  Ground_1 : Ground
  Ground_2 : Ground
  Terminator_1 : Terminator
  OpStatus : ArgIn  OutDataTypeStr=Enum: Dcm_OpStatusType  ArgumentName=OpStatus
  ErrorCode : ArgOut  OutDataTypeStr=Enum: Dcm_NegativeResponseCodeType  ArgumentName=ErrorCode
  ERR : ArgOut  Port=2  OutDataTypeStr=Std_ReturnType  ArgumentName=ERR
  wires:
  OpStatus/1 -> Terminator_1/1
  Ground_1/1 -> ErrorCode/1
  Ground_2/1 -> ERR/1

== system cddDcmApp/DataServices_DID_9809_3_actuator_positionSecond_temperature_actuator_position/DataServices_DID_9809_3_actuator_positionSecond_temperature_actuator_position_ReadData_sys ==
  function : TriggerPort  TriggerType=function-call  IsSimulinkFunction=on  FunctionName=DataServices_DID_9809_3_actuator_positionSecond_temperature_actuator_position_ReadData  VariantControl=(inherit)
  Ground_1 : Ground
  Ground_2 : Ground
  Terminator_1 : Terminator
  OpStatus : ArgIn  OutDataTypeStr=Enum: Dcm_OpStatusType  ArgumentName=OpStatus
  Data : ArgOut  OutDataTypeStr=uint8  ArgumentName=Data
  ERR : ArgOut  Port=2  OutDataTypeStr=Std_ReturnType  ArgumentName=ERR
  wires:
  OpStatus/1 -> Terminator_1/1
  Ground_1/1 -> Data/1
  Ground_2/1 -> ERR/1

== system cddDcmApp/DataServices_DID_9809_3_actuator_positionSecond_temperature_actuator_position/DataServices_DID_9809_3_actuator_positionSecond_temperature_actuator_position_ReturnControlToECU_sys ==
  function : TriggerPort  TriggerType=function-call  IsSimulinkFunction=on  FunctionName=DataServices_DID_9809_3_actuator_positionSecond_temperature_actuator_position_ReturnControlToECU  VariantControl=(inherit)
  Ground_1 : Ground
  Ground_2 : Ground
  ErrorCode : ArgOut  OutDataTypeStr=Enum: Dcm_NegativeResponseCodeType  ArgumentName=ErrorCode
  ERR : ArgOut  Port=2  OutDataTypeStr=Std_ReturnType  ArgumentName=ERR
  wires:
  Ground_1/1 -> ErrorCode/1
  Ground_2/1 -> ERR/1

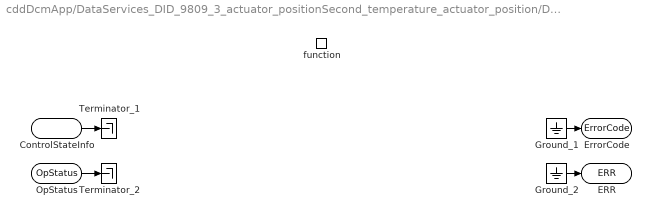
== system cddDcmApp/DataServices_DID_9809_3_actuator_positionSecond_temperature_actuator_position/DataServices_DID_9809_3_actuator_positionSecond_temperature_actuator_position_ShortTermAdjustment_sys ==
  function : TriggerPort  TriggerType=function-call  IsSimulinkFunction=on  FunctionName=DataServices_DID_9809_3_actuator_positionSecond_temperature_actuator_position_ShortTermAdjustment  VariantControl=(inherit)
  Ground_1 : Ground
  Ground_2 : Ground
  Terminator_1 : Terminator
  Terminator_2 : Terminator
  ControlStateInfo : ArgIn  OutDataTypeStr=uint8  ArgumentName=ControlStateInfo
  OpStatus : ArgIn  Port=2  OutDataTypeStr=Enum: Dcm_OpStatusType  ArgumentName=OpStatus
  ErrorCode : ArgOut  OutDataTypeStr=Enum: Dcm_NegativeResponseCodeType  ArgumentName=ErrorCode
  ERR : ArgOut  Port=2  OutDataTypeStr=Std_ReturnType  ArgumentName=ERR
  wires:
  ControlStateInfo/1 -> Terminator_1/1
  OpStatus/1 -> Terminator_2/1
  Ground_1/1 -> ErrorCode/1
  Ground_2/1 -> ERR/1

== system cddDcmApp/DataServices_DID_9809_DID_9809_1_Front_left_temperature_actuator_position/DataServices_DID_9809_DID_9809_1_Front_left_temperature_actuator_position_FreezeCurrentState_sys ==
  function : TriggerPort  TriggerType=function-call  IsSimulinkFunction=on  FunctionName=DataServices_DID_9809_DID_9809_1_Front_left_temperature_actuator_position_FreezeCurrentState  VariantControl=(inherit)
  Ground_1 : Ground
  Ground_2 : Ground
  Terminator_1 : Terminator
  OpStatus : ArgIn  OutDataTypeStr=Enum: Dcm_OpStatusType  ArgumentName=OpStatus
  ErrorCode : ArgOut  OutDataTypeStr=Enum: Dcm_NegativeResponseCodeType  ArgumentName=ErrorCode
  ERR : ArgOut  Port=2  OutDataTypeStr=Std_ReturnType  ArgumentName=ERR
  wires:
  OpStatus/1 -> Terminator_1/1
  Ground_1/1 -> ErrorCode/1
  Ground_2/1 -> ERR/1

== system cddDcmApp/DataServices_DID_9809_DID_9809_1_Front_left_temperature_actuator_position/DataServices_DID_9809_DID_9809_1_Front_left_temperature_actuator_position_ReadData_sys ==
  function : TriggerPort  TriggerType=function-call  IsSimulinkFunction=on  FunctionName=DataServices_DID_9809_DID_9809_1_Front_left_temperature_actuator_position_ReadData  VariantControl=(inherit)
  Ground_1 : Ground
  Ground_2 : Ground
  Terminator_1 : Terminator
  OpStatus : ArgIn  OutDataTypeStr=Enum: Dcm_OpStatusType  ArgumentName=OpStatus
  Data : ArgOut  OutDataTypeStr=uint8  ArgumentName=Data
  ERR : ArgOut  Port=2  OutDataTypeStr=Std_ReturnType  ArgumentName=ERR
  wires:
  OpStatus/1 -> Terminator_1/1
  Ground_1/1 -> Data/1
  Ground_2/1 -> ERR/1

== system cddDcmApp/DataServices_DID_9809_DID_9809_1_Front_left_temperature_actuator_position/DataServices_DID_9809_DID_9809_1_Front_left_temperature_actuator_position_ReturnControlToECU_sys ==
  function : TriggerPort  TriggerType=function-call  IsSimulinkFunction=on  FunctionName=DataServices_DID_9809_DID_9809_1_Front_left_temperature_actuator_position_ReturnControlToECU  VariantControl=(inherit)
  Ground_1 : Ground
  Ground_2 : Ground
  ErrorCode : ArgOut  OutDataTypeStr=Enum: Dcm_NegativeResponseCodeType  ArgumentName=ErrorCode
  ERR : ArgOut  Port=2  OutDataTypeStr=Std_ReturnType  ArgumentName=ERR
  wires:
  Ground_1/1 -> ErrorCode/1
  Ground_2/1 -> ERR/1

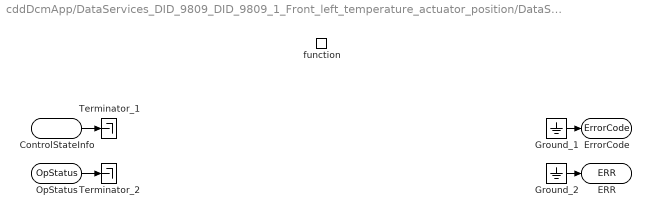
== system cddDcmApp/DataServices_DID_9809_DID_9809_1_Front_left_temperature_actuator_position/DataServices_DID_9809_DID_9809_1_Front_left_temperature_actuator_position_ShortTermAdjustment_sys ==
  function : TriggerPort  TriggerType=function-call  IsSimulinkFunction=on  FunctionName=DataServices_DID_9809_DID_9809_1_Front_left_temperature_actuator_position_ShortTermAdjustment  VariantControl=(inherit)
  Ground_1 : Ground
  Ground_2 : Ground
  Terminator_1 : Terminator
  Terminator_2 : Terminator
  ControlStateInfo : ArgIn  OutDataTypeStr=uint8  ArgumentName=ControlStateInfo
  OpStatus : ArgIn  Port=2  OutDataTypeStr=Enum: Dcm_OpStatusType  ArgumentName=OpStatus
  ErrorCode : ArgOut  OutDataTypeStr=Enum: Dcm_NegativeResponseCodeType  ArgumentName=ErrorCode
  ERR : ArgOut  Port=2  OutDataTypeStr=Std_ReturnType  ArgumentName=ERR
  wires:
  ControlStateInfo/1 -> Terminator_1/1
  OpStatus/1 -> Terminator_2/1
  Ground_1/1 -> ErrorCode/1
  Ground_2/1 -> ERR/1

== system cddDcmApp/DataServices_DID_9809_DID_9809_2_Front_right_temperature_actuator_position/DataServices_DID_9809_DID_9809_2_Front_right_temperature_actuator_position_FreezeCurrentState_sys ==
  function : TriggerPort  TriggerType=function-call  IsSimulinkFunction=on  FunctionName=DataServices_DID_9809_DID_9809_2_Front_right_temperature_actuator_position_FreezeCurrentState  VariantControl=(inherit)
  Ground_1 : Ground
  Ground_2 : Ground
  Terminator_1 : Terminator
  OpStatus : ArgIn  OutDataTypeStr=Enum: Dcm_OpStatusType  ArgumentName=OpStatus
  ErrorCode : ArgOut  OutDataTypeStr=Enum: Dcm_NegativeResponseCodeType  ArgumentName=ErrorCode
  ERR : ArgOut  Port=2  OutDataTypeStr=Std_ReturnType  ArgumentName=ERR
  wires:
  OpStatus/1 -> Terminator_1/1
  Ground_1/1 -> ErrorCode/1
  Ground_2/1 -> ERR/1

== system cddDcmApp/DataServices_DID_9809_DID_9809_2_Front_right_temperature_actuator_position/DataServices_DID_9809_DID_9809_2_Front_right_temperature_actuator_position_ReadData_sys ==
  function : TriggerPort  TriggerType=function-call  IsSimulinkFunction=on  FunctionName=DataServices_DID_9809_DID_9809_2_Front_right_temperature_actuator_position_ReadData  VariantControl=(inherit)
  Ground_1 : Ground
  Ground_2 : Ground
  Terminator_1 : Terminator
  OpStatus : ArgIn  OutDataTypeStr=Enum: Dcm_OpStatusType  ArgumentName=OpStatus
  Data : ArgOut  OutDataTypeStr=uint8  ArgumentName=Data
  ERR : ArgOut  Port=2  OutDataTypeStr=Std_ReturnType  ArgumentName=ERR
  wires:
  OpStatus/1 -> Terminator_1/1
  Ground_1/1 -> Data/1
  Ground_2/1 -> ERR/1

== system cddDcmApp/DataServices_DID_9809_DID_9809_2_Front_right_temperature_actuator_position/DataServices_DID_9809_DID_9809_2_Front_right_temperature_actuator_position_ReturnControlToECU_sys ==
  function : TriggerPort  TriggerType=function-call  IsSimulinkFunction=on  FunctionName=DataServices_DID_9809_DID_9809_2_Front_right_temperature_actuator_position_ReturnControlToECU  VariantControl=(inherit)
  Ground_1 : Ground
  Ground_2 : Ground
  ErrorCode : ArgOut  OutDataTypeStr=Enum: Dcm_NegativeResponseCodeType  ArgumentName=ErrorCode
  ERR : ArgOut  Port=2  OutDataTypeStr=Std_ReturnType  ArgumentName=ERR
  wires:
  Ground_1/1 -> ErrorCode/1
  Ground_2/1 -> ERR/1

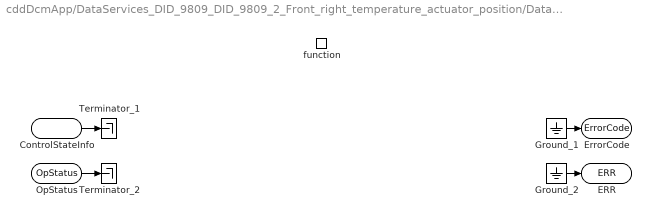
== system cddDcmApp/DataServices_DID_9809_DID_9809_2_Front_right_temperature_actuator_position/DataServices_DID_9809_DID_9809_2_Front_right_temperature_actuator_position_ShortTermAdjustment_sys ==
  function : TriggerPort  TriggerType=function-call  IsSimulinkFunction=on  FunctionName=DataServices_DID_9809_DID_9809_2_Front_right_temperature_actuator_position_ShortTermAdjustment  VariantControl=(inherit)
  Ground_1 : Ground
  Ground_2 : Ground
  Terminator_1 : Terminator
  Terminator_2 : Terminator
  ControlStateInfo : ArgIn  OutDataTypeStr=uint8  ArgumentName=ControlStateInfo
  OpStatus : ArgIn  Port=2  OutDataTypeStr=Enum: Dcm_OpStatusType  ArgumentName=OpStatus
  ErrorCode : ArgOut  OutDataTypeStr=Enum: Dcm_NegativeResponseCodeType  ArgumentName=ErrorCode
  ERR : ArgOut  Port=2  OutDataTypeStr=Std_ReturnType  ArgumentName=ERR
  wires:
  ControlStateInfo/1 -> Terminator_1/1
  OpStatus/1 -> Terminator_2/1
  Ground_1/1 -> ErrorCode/1
  Ground_2/1 -> ERR/1

== system cddDcmApp/DataServices_DID_9809_DID_9809_4_Second_right_temperature_actuator_position/DataServices_DID_9809_DID_9809_4_Second_right_temperature_actuator_position_FreezeCurrentState_sys ==
  function : TriggerPort  TriggerType=function-call  IsSimulinkFunction=on  FunctionName=DataServices_DID_9809_DID_9809_4_Second_right_temperature_actuator_position_FreezeCurrentState  VariantControl=(inherit)
  Ground_1 : Ground
  Ground_2 : Ground
  Terminator_1 : Terminator
  OpStatus : ArgIn  OutDataTypeStr=Enum: Dcm_OpStatusType  ArgumentName=OpStatus
  ErrorCode : ArgOut  OutDataTypeStr=Enum: Dcm_NegativeResponseCodeType  ArgumentName=ErrorCode
  ERR : ArgOut  Port=2  OutDataTypeStr=Std_ReturnType  ArgumentName=ERR
  wires:
  OpStatus/1 -> Terminator_1/1
  Ground_1/1 -> ErrorCode/1
  Ground_2/1 -> ERR/1

== system cddDcmApp/DataServices_DID_9809_DID_9809_4_Second_right_temperature_actuator_position/DataServices_DID_9809_DID_9809_4_Second_right_temperature_actuator_position_ReadData_sys ==
  function : TriggerPort  TriggerType=function-call  IsSimulinkFunction=on  FunctionName=DataServices_DID_9809_DID_9809_4_Second_right_temperature_actuator_position_ReadData  VariantControl=(inherit)
  Ground_1 : Ground
  Ground_2 : Ground
  Terminator_1 : Terminator
  OpStatus : ArgIn  OutDataTypeStr=Enum: Dcm_OpStatusType  ArgumentName=OpStatus
  Data : ArgOut  OutDataTypeStr=uint8  ArgumentName=Data
  ERR : ArgOut  Port=2  OutDataTypeStr=Std_ReturnType  ArgumentName=ERR
  wires:
  OpStatus/1 -> Terminator_1/1
  Ground_1/1 -> Data/1
  Ground_2/1 -> ERR/1

== system cddDcmApp/DataServices_DID_9809_DID_9809_4_Second_right_temperature_actuator_position/DataServices_DID_9809_DID_9809_4_Second_right_temperature_actuator_position_ReturnControlToECU_sys ==
  function : TriggerPort  TriggerType=function-call  IsSimulinkFunction=on  FunctionName=DataServices_DID_9809_DID_9809_4_Second_right_temperature_actuator_position_ReturnControlToECU  VariantControl=(inherit)
  Ground_1 : Ground
  Ground_2 : Ground
  ErrorCode : ArgOut  OutDataTypeStr=Enum: Dcm_NegativeResponseCodeType  ArgumentName=ErrorCode
  ERR : ArgOut  Port=2  OutDataTypeStr=Std_ReturnType  ArgumentName=ERR
  wires:
  Ground_1/1 -> ErrorCode/1
  Ground_2/1 -> ERR/1

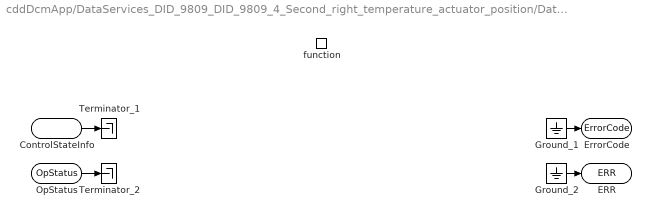
== system cddDcmApp/DataServices_DID_9809_DID_9809_4_Second_right_temperature_actuator_position/DataServices_DID_9809_DID_9809_4_Second_right_temperature_actuator_position_ShortTermAdjustment_sys ==
  function : TriggerPort  TriggerType=function-call  IsSimulinkFunction=on  FunctionName=DataServices_DID_9809_DID_9809_4_Second_right_temperature_actuator_position_ShortTermAdjustment  VariantControl=(inherit)
  Ground_1 : Ground
  Ground_2 : Ground
  Terminator_1 : Terminator
  Terminator_2 : Terminator
  ControlStateInfo : ArgIn  OutDataTypeStr=uint8  ArgumentName=ControlStateInfo
  OpStatus : ArgIn  Port=2  OutDataTypeStr=Enum: Dcm_OpStatusType  ArgumentName=OpStatus
  ErrorCode : ArgOut  OutDataTypeStr=Enum: Dcm_NegativeResponseCodeType  ArgumentName=ErrorCode
  ERR : ArgOut  Port=2  OutDataTypeStr=Std_ReturnType  ArgumentName=ERR
  wires:
  ControlStateInfo/1 -> Terminator_1/1
  OpStatus/1 -> Terminator_2/1
  Ground_1/1 -> ErrorCode/1
  Ground_2/1 -> ERR/1
